simulink model "r2_ratty2_32k_r203"  (R2012b)
settings: Solver=ode45  StartTime=0.0  StopTime=10.0  RelTol=1e-3
undefined symbols (not defined in the file): Down, pfb_coeff_gen_calc, Up
libraries: xbsIndex_r4 x20024, casper_library_misc x89, casper_library_delays x41, xps_library x14, xbsReplacements_r4 x2, simulink x1, casper_library_scopes x1
(part 135 of 207)

== system r2_ratty2_32k_r203/fft/fft_direct/butterfly2_3/twiddle/coeff_gen/cosin ==
  theta : Inport
  misci : Inport  Port=2
  Constant4 : xbsIndex_r4/Constant  const=0  gui_display_data_type=Fixed-point  arith_type=Unsigned  n_bits=1  bin_pt=0  preci_type=Single  exp_width=8  frac_width=24  explicit_period=on  period=1  equ=P=C  opselect=C  inp2=PCIN>>17  opr=+  inp1=P  carry=CIN  dbl_ovrd=off  block_type=constant
  Constant5 : xbsIndex_r4/Constant  const=0  gui_display_data_type=Fixed-point  arith_type=Unsigned  n_bits=1  bin_pt=0  preci_type=Single  exp_width=8  frac_width=24  explicit_period=on  period=1  equ=P=C  opselect=C  inp2=PCIN>>17  opr=+  inp1=P  carry=CIN  dbl_ovrd=off  block_type=constant
  Delay : xbsIndex_r4/Delay  rst=off  infoeditControl=Selection of Reset will increase slice count due to use of real FFs and instead of SRLs  en=off  latency=bram_latency  dbl_ovrd=off  reg_retiming=on  block_type=delay
  Delay10 : xbsIndex_r4/Delay  rst=off  infoeditControl=Selection of Reset will increase slice count due to use of real FFs and instead of SRLs  en=off  latency=bram_latency  dbl_ovrd=off  reg_retiming=on  block_type=delay
  Delay8 : xbsIndex_r4/Delay  rst=off  infoeditControl=Selection of Reset will increase slice count due to use of real FFs and instead of SRLs  en=off  latency=bram_latency  dbl_ovrd=off  reg_retiming=on  block_type=delay
  ROM : xbsIndex_r4/ROM  depth=2^(13)  initVector=[44423615990 44421256703 44418897416 44416538129 44414440986 44412081699 44409722411 44407363124 44405003837 44402644550 44400285263 44397925976 44395828833 44393469546 44391110259 44388750971 44386391684 44384032397 44381673110 44379313823 44377216680 44374857393 44372498106 44370138819 44367779532 44365420245 44363060957 44360701670 44358604527 44356245240 44353885953 44351526666 44349167379 44346808092 44344448805 44342351662 44339992375 44337633087 44335273800 44332914513 44330555226 44328458083 44326098796 44323739509 44321380222 44319020935 44316661648 44314302361 44312205218 44309845931 44307486643 44305127356 44302768069 44300408782 44298311639 44295952352 44293593065 44291233778 44288874491 44286515204 44284155917 44282058774 44279699487 44277340200 44274980913 44272621625 44270262338 44268165195 44265805908 44263446621 44261087334 44258728047 44256630904 44254271617 44251912330 44249553043 44247193756 44244834469 44242737326 44240378039 44238018752 44235659465 44233300178 44231203035 44228843748 44226484460 44224125173 44221765886 44219406599 44217309456 44214950169 44212590882 44210231595 44207872308 44205775165 44203415878 44201056591 44198697304 44196338017 44194240874 44191881587 44189522300 44187163013 44184803726 44182706583 44180347296 44177988009 44175628722 44173269435 44171172292 44168813005 44166453718 44164094431 44161735144 44159638001 44157278714 44154919427 44152560140 44150462997 44148103710 44145744423 44143385135 44141025848 44138928705 44136569418 44134210131 44131850844 44129753701 44127394414 44125035127 44122675840 44120316553 44118219410 44115860123 44113500836 44111141549 44109044406 44106685119 44104325832 44101966545 44099607258 44097510115 44095150828 44092791541 44090432254 44088335111 44085975824 44083616537 44081257250 44079160107 44076800821 44074441534 44072082247 44069985104 44067625817 44065266530 44062907243 44060810100 44058450813 44056091526 44053732239 44051635096 44049275809 44046916522 44044557235 44042460092 44040100805 44037741518 44035382231 44033285088 44030925801 44028566514 44026207227 44024110084 44021750797 44019391510 44017032223 44014935080 44012575793 44010216506 44008119363 44005760076 44003400789 44001041502 43998944359 43996585072 43994225786 43991866499 43989769356 43987410069 43985050782 43982953639 43980594352 43978235065 43975875778 43973778635 43971419348 43969060061 43966962918 43964603631 43962244344 43959885057 43957787914 43955428627 43953069340 43950972197 43948612911 43946253624 43943894337 43941797194 43939437907 43937078620 43934981477 43932622190 43930262903 43927903616 43925806473 43923447186 43921087899 43918990756 43916631469 43914272183 43912175040 43909815753 43907456466 43905359323 43903000036 43900640749 43898281462 43896184319 43893825032 43891465745 43889368602 43887009315 43884650029 43882552886 43880193599 43877834312 43875737169 43873377882 43871018595 43868921452 43866562165 43864202878 43862105735 43859746448 43857387162 43855290019 43852930732 43850571445 43848212158 43846115015 43843755728 43841396441 43839299298 43836940011 43834580725 43832483582 43830124295 43827765008 43825667865 43823308578 43821211435 43818852148 43816492861 43814395718 43812036432 43809677145 43807580002 43805220715 43802861428 43800764285 43798404998 43796045711 43793948568 43791589282 43789229995 43787132852 43784773565 43782414278 43780317135 43777957848 43775598561 43773501419 43771142132 43769044989 43766685702 43764326415 43762229272 43759869985 43757510698 43755413556 43753054269 43750694982 43748597839 43746238552 43744141409 43741782122 43739422836 43737325693 43734966406 43732607119 43730509976 43728150689 43726053546 43723694259 43721334973 43719237830 43716878543 43714519256 43712422113 43710062826 43707965684 43705606397 43703247110 43701149967 43698790680 43696693537 43694334250 43691974964 43689877821 43687518534 43685159247 43683062104 43680702817 43678605674 43676246388 43673887101 43671789958 43669430671 43667333528 43664974241 43662614955 ...(+94308 chars)]  distributed_mem=Block RAM  rst=off  init_reg=0  en=off  latency=bram_latency  gui_display_data_type=Fixed-point  arith_type=Unsigned  n_bits=n_bits*2  bin_pt=0  preci_type=Single  exp_width=8  frac_width=24  dbl_ovrd=off  optimize=Speed  use_rpm=on  block_type=sprom
  Terminator : Terminator
  Terminator1 : Terminator
  Terminator4 : Terminator
  add_convert0 : Subsystem
  add_convert1 : Subsystem
  assert : xbsIndex_r4/Assert  assert_type=on  type_source=Explicitly  gui_display_data_type=Fixed-point  arith_type=Unsigned  n_bits=13  bin_pt=0  preci_type=Single  exp_width=8  frac_width=24  assert_rate=off  rate_source=Explicitly  period=1  output_port=on  block_type=assert
  c_to_ri : casper_library_misc/c_to_ri  n_bits=n_bits  bin_pt=bin_pt
  invert0 : Subsystem  mask{n_bits=n_bits, bin_pt=bin_pt, neg_latency=neg_latency, mux_latency=mux_latency}
      mask prompts: n_bits "bit width"; bin_pt "binary point"; neg_latency "negate latency"; mux_latency "mux latency"
  invert1 : Subsystem  mask{n_bits=n_bits, bin_pt=bin_pt, neg_latency=neg_latency, mux_latency=mux_latency}
      mask prompts: n_bits "bit width"; bin_pt "binary point"; neg_latency "negate latency"; mux_latency "mux latency"
  -sin : Outport
  -cos : Outport  Port=2
  misco : Outport  Port=3
  wires:
  theta/1 -> assert/1
  assert/1 -> add_convert0/1(theta), add_convert1/1(theta)
  Constant4/1 -> add_convert0/2(misci)
  add_convert0/3(misco) -> Terminator/1
  misci/1 -> add_convert1/2(misci)
  add_convert1/3(misco) -> Delay/1
  add_convert0/2(add) -> ROM/1
  add_convert1/2(add) -> Terminator4/1
  ROM/1 -> c_to_ri/1
  add_convert1/1(negate) -> Delay8/1
  add_convert0/1(negate) -> Delay10/1
  Delay10/1 -> invert0/1(negate)
  Constant5/1 -> invert0/3(misci)
  c_to_ri/1 -> invert0/2(in)
  Delay8/1 -> invert1/1(negate)
  c_to_ri/2 -> invert1/2(in)
  invert0/2(misco) -> Terminator1/1
  Delay/1 -> invert1/3(misci)
  invert0/1(out) -> -sin/1
  invert1/2(misco) -> misco/1
  invert1/1(out) -> -cos/1
